simulink model "TosAeb"  (R2021a)
settings: Solver=FixedStepDiscrete  StartTime=0.0  StopTime=inf  FixedStep=0.01  RelTol=1e-3
undefined symbols (not defined in the file or in its folder): ACCModSts, AccSts1, AEB_ObjectMovingOrientation, AEB_ObjectMovingState, CN_BTCActive, CN_CurrentGearR, CN_CurrentGearR_DHT, CN_PTCActive, CN_VDCActive, CN_VSC_EspOff, CN_VSC_FCW_AEB_Error, CN_VSC_FCW_AEB_NoDispay, CN_VSC_FCW_AEB_PerfmDgdtn, CN_VSC_FCW_AEB_TempUnAvlbl, CN_VSC_VehStandstill, CNaccelAvoidMax, CNaccelAvoidMin, CNbrakePedalPress, CNcomfortStrLeftAccelMax, CNcomfortStrLeftAccelMin, CNcomfortStrRightAccelMax, CNcomfortStrRightAccelMin, CNDrvDoorOpen, CNDrvModeEco, CNDrvModeNorm, CNDrvModeOther, CNDrvModeSport, CNDrvSBRreminder, CNNull, CNobjInvalidID, CNPassDoorOpen, CNPassSBRreminder, CNPEBSAccelNegInf, CNPEBSAccelNull, CNPEBSTimeInf, CNPEBSTimeNull, CNSTOPMAX, CNSTOPNULL, CNsubjWidth, CNSwtOFF, CNSwtON, CNTTCMAX, CNTTCNULL, CNVehConfigAEBCycNone, CNVehConfigAEBNone, CNVehConfigAEBPedNone, CNVehConfigFCWNone, CNVehConfigJANone, CollisionSeverity, FALSE, FCWSnvty, JACycObjCalcInfoDefaultParams, JAPedObjCalcInfoDefaultParams, JAVehObjCalcInfoDefaultParams, latCycObjCalcInfoDefaultParams, latPedObjCalcInfoDefaultParams, longObjCalcInfoDefaultParams, ModSts, movingStatus, ObjectType ... (+1112)
libraries: simulink x3031, AEBSFunctionLib x50, Ta_Library x33, AEBSStateMachineLib x23, Mylibrary x12, simulink_extras x7, ObjSelect_lib x3, dspstat3 x2
(part 80 of 102)

== system TosAeb/FcwAebJa_Step_sys/ALG_AEBSystem/ALG_AEBController/AEB_LongTgtInfoCalc/LongObjCalcInfoCalc/AEBS_LongCollisionAssesApp/collisionSeverityHighCalc/collisionSeverityHighCalc1/CollisionAssessHighCalcVersion3/CollisionAssessHighCalc1/CalcBrakePhase3/BrkSysRuntime ==
  a0Obj : Inport
  accelMaxDec : Inport  Port=2
  v0Obj : Inport  Port=3
  v0Ego : Inport  Port=4
  Bus Creator : BusCreator  Inputs=4
  Bus Selector : BusSelector  OutputSignals=a0Obj,accelMaxDec
  Bus Selector1 : BusSelector  OutputSignals=v0Ego,accelMaxDec
  Bus Selector2 : BusSelector  OutputSignals=v0Ego,accelMaxDec,v0Obj,a0Obj
  If : If  IfExpression=u1
  Merge : Merge
  Subsystem : Subsystem
  a0ObjGreatSituation : Subsystem  TreatAsAtomicUnit=on
  a0ObjLessSituation : Subsystem  TreatAsAtomicUnit=on
  tTime : Outport
  wires:
  [a0ObjGreat] -> a0ObjGreatSituation/Action
  If/2 -> [a0ObjGreat]
  Bus Selector2/4 -> a0ObjGreatSituation/4(a0Obj)  "<a0Obj>"
  Bus Selector2/3 -> a0ObjGreatSituation/3(v0Obj)  "<v0Obj>"
  Bus Selector2/2 -> a0ObjGreatSituation/2(accelMaxDec)  "<accelMaxDec>"
  Bus Selector2/1 -> a0ObjGreatSituation/1(vtEgo)  "<v0Ego>"
  Bus Selector1/2 -> a0ObjLessSituation/2(accelMaxDec)  "<accelMaxDec>"
  Bus Selector1/1 -> a0ObjLessSituation/1(vtEgo)  "<v0Ego>"
  Bus Creator/1 -> Bus Selector/1, Bus Selector1/1, Bus Selector2/1
  Bus Selector/2 -> Subsystem/2(accelMaxDec)  "<accelMaxDec>"
  Bus Selector/1 -> Subsystem/1(a0Obj)  "<a0Obj>"
  v0Obj/1 -> Bus Creator/4  "v0Obj"
  v0Ego/1 -> Bus Creator/3  "v0Ego"
  accelMaxDec/1 -> Bus Creator/2  "accelMaxDec"
  Subsystem/1(Flg) -> If/1
  a0Obj/1 -> Bus Creator/1  "a0Obj"
  If/1 -> a0ObjLessSituation/Action
  a0ObjLessSituation/1(tTime) -> Merge/1
  a0ObjGreatSituation/1(tTime) -> Merge/2
  Merge/1 -> tTime/1

== system TosAeb/FcwAebJa_Step_sys/ALG_AEBSystem/ALG_AEBController/AEB_LongTgtInfoCalc/LongObjCalcInfoCalc/AEBS_LongCollisionAssesApp/collisionSeverityHighCalc/collisionSeverityHighCalc1/CollisionAssessHighCalcVersion3/CollisionAssessHighCalc1/CalcBrakePhase3/egoCollisionFlgCalc ==
  stEgo : Inport
  stObj : Inport  Port=2
  dx0 : Inport  Port=3
  Add : Sum
  Compare To Constant : simulink/Logic and Bit Operations/Compare To Constant  relop=<  const=0  OutDataTypeStr=boolean
  Subtract : Sum  Inputs=+-
  egoCollisionFlg : Outport
  wires:
  stObj/1 -> Add/1
  dx0/1 -> Add/2
  Add/1 -> Subtract/1
  stEgo/1 -> Subtract/2
  Subtract/1 -> Compare To Constant/1
  Compare To Constant/1 -> egoCollisionFlg/1

== system TosAeb/FcwAebJa_Step_sys/ALG_AEBSystem/ALG_AEBController/AEB_LongTgtInfoCalc/LongObjCalcInfoCalc/AEBS_LongCollisionAssesApp/collisionSeverityHighCalc/collisionSeverityHighCalc1/CollisionAssessHighCalcVersion3/CollisionAssessHighCalc1/CalcBrakePhase3/stEgoCalc ==
  tTime : Inport
  v0 : Inport  Port=2
  a0 : Inport  Port=3
  Add1 : Sum  Inputs=2
  Constant1 : Constant  Value=0.5
  Product1 : Product  Inputs=4
  Product4 : Product
  st : Outport
  wires:
  tTime/1 -> Product1/4, Product4/1, Product1/3
  Constant1/1 -> Product1/1
  a0/1 -> Product1/2
  v0/1 -> Product4/2
  Product1/1 -> Add1/1
  Product4/1 -> Add1/2
  Add1/1 -> st/1

== system TosAeb/FcwAebJa_Step_sys/ALG_AEBSystem/ALG_AEBController/AEB_LongTgtInfoCalc/LongObjCalcInfoCalc/AEBS_LongCollisionAssesApp/collisionSeverityHighCalc/collisionSeverityHighCalc1/CollisionAssessHighCalcVersion3/CollisionAssessHighCalc1/CalcBrakePhase3/stObjCalc ==
  tTime : Inport
  a0 : Inport  Port=2
  v0 : Inport  Port=3
  Add1 : Sum  Inputs=2
  Constant1 : Constant  Value=0.5
  Product1 : Product  Inputs=4
  Product4 : Product
  st : Outport
  wires:
  Add1/1 -> st/1
  Product4/1 -> Add1/2
  Product1/1 -> Add1/1
  v0/1 -> Product4/2
  a0/1 -> Product1/2
  Constant1/1 -> Product1/1
  tTime/1 -> Product4/1, Product1/4, Product1/3

== system TosAeb/FcwAebJa_Step_sys/ALG_AEBSystem/ALG_AEBController/AEB_LongTgtInfoCalc/LongObjCalcInfoCalc/AEBS_LongCollisionAssesApp/collisionSeverityHighCalc/collisionSeverityHighCalc1/CollisionAssessHighCalcVersion3/CollisionAssessHighCalc1/CalcBrakePhase3/tEgoStopCalc ==
  a0Ego : Inport
  v0Ego : Inport  Port=2
  tStopCalc : Subsystem  TreatAsAtomicUnit=on
  tEgoStop : Outport
  wires:
  v0Ego/1 -> tStopCalc/2(velAbs)
  a0Ego/1 -> tStopCalc/1(accelAbs)
  tStopCalc/1(tStop) -> tEgoStop/1

== system TosAeb/FcwAebJa_Step_sys/ALG_AEBSystem/ALG_AEBController/AEB_LongTgtInfoCalc/LongObjCalcInfoCalc/AEBS_LongCollisionAssesApp/collisionSeverityHighCalc/collisionSeverityHighCalc1/CollisionAssessHighCalcVersion3/CollisionAssessHighCalc1/CalcBrakePhase3/tObjRunCalc ==
  tEgoRun : Inport
  tObjStop : Inport  Port=2
  MinMax : MinMax  Inputs=2
  tObjRun : Outport
  wires:
  MinMax/1 -> tObjRun/1
  tEgoRun/1 -> MinMax/1
  tObjStop/1 -> MinMax/2

== system TosAeb/FcwAebJa_Step_sys/ALG_AEBSystem/ALG_AEBController/AEB_LongTgtInfoCalc/LongObjCalcInfoCalc/AEBS_LongCollisionAssesApp/collisionSeverityHighCalc/collisionSeverityHighCalc1/CollisionAssessHighCalcVersion3/CollisionAssessHighCalc1/CalcBrakePhase3/tObjStopCalc ==
  a0Obj : Inport
  v0Obj : Inport  Port=2
  tStopCalc : Subsystem  TreatAsAtomicUnit=on
  tObjStop : Outport
  wires:
  v0Obj/1 -> tStopCalc/2(velAbs)
  a0Obj/1 -> tStopCalc/1(accelAbs)
  tStopCalc/1(tStop) -> tObjStop/1

== system TosAeb/FcwAebJa_Step_sys/ALG_AEBSystem/ALG_AEBController/AEB_LongTgtInfoCalc/LongObjCalcInfoCalc/AEBS_LongCollisionAssesApp/collisionSeverityHighCalc/collisionSeverityHighCalc1/CollisionAssessHighCalcVersion3/CollisionAssessHighCalc1/CalcBrakePhase3/time4EgoPredic ==
  tEgoStop : Inport
  tEgovDelata : Inport  Port=2
  tTime : Inport  Port=3
  MinMax : MinMax  Inputs=3
  tEgoRun : Outport
  wires:
  tTime/1 -> MinMax/3
  tEgoStop/1 -> MinMax/1
  MinMax/1 -> tEgoRun/1
  tEgovDelata/1 -> MinMax/2

== system TosAeb/FcwAebJa_Step_sys/ALG_AEBSystem/ALG_AEBController/AEB_LongTgtInfoCalc/LongObjCalcInfoCalc/AEBS_LongCollisionAssesApp/collisionSeverityHighCalc/collisionSeverityHighCalc1/CollisionAssessHighCalcVersion3/CollisionAssessHighCalc1/CalcBrakePhase3/timeEgovDeltaCalc ==
  v0Ego : Inport
  velMaxReudce : Inport  Port=2
  a0Ego : Inport  Port=3
  timeEgovDelata : Subsystem  TreatAsAtomicUnit=on
  velMaxReudceFiltCalc : Subsystem
  tEgovDelata : Outport
  wires:
  timeEgovDelata/1(tStop) -> tEgovDelata/1
  a0Ego/1 -> timeEgovDelata/1(accelAbs)
  velMaxReudceFiltCalc/1(velMaxReudceFilt) -> timeEgovDelata/2(velAbs)
  velMaxReudce/1 -> velMaxReudceFiltCalc/2(velMaxReudce)
  v0Ego/1 -> velMaxReudceFiltCalc/1(v0Ego)

== system TosAeb/FcwAebJa_Step_sys/ALG_AEBSystem/ALG_AEBController/AEB_LongTgtInfoCalc/LongObjCalcInfoCalc/AEBS_LongCollisionAssesApp/collisionSeverityHighCalc/collisionSeverityHighCalc1/CollisionAssessHighCalcVersion3/CollisionAssessHighCalc2/CalcBrakePhase1/egoCollisionFlgCalc ==
  stEgo : Inport
  stObj : Inport  Port=2
  dx : Inport  Port=3
  Add : Sum
  Compare To Constant : simulink/Logic and Bit Operations/Compare To Constant  relop=<  const=0  OutDataTypeStr=boolean
  Subtract : Sum  Inputs=+-
  egoCollisionFlg : Outport
  wires:
  stObj/1 -> Add/1
  dx/1 -> Add/2
  Add/1 -> Subtract/1
  stEgo/1 -> Subtract/2
  Subtract/1 -> Compare To Constant/1
  Compare To Constant/1 -> egoCollisionFlg/1

== system TosAeb/FcwAebJa_Step_sys/ALG_AEBSystem/ALG_AEBController/AEB_LongTgtInfoCalc/LongObjCalcInfoCalc/AEBS_LongCollisionAssesApp/collisionSeverityHighCalc/collisionSeverityHighCalc1/CollisionAssessHighCalcVersion3/CollisionAssessHighCalc2/CalcBrakePhase1/restDeltaVelCalc ==
  velMaxReudce : Inport
  v0 : Inport  Port=2
  vt : Inport  Port=3
  Subtract : Sum  Inputs=+-
  Subtract1 : Sum  Inputs=+-
  restDeltaVel : Outport
  wires:
  v0/1 -> Subtract/1
  vt/1 -> Subtract/2
  Subtract/1 -> Subtract1/2
  velMaxReudce/1 -> Subtract1/1
  Subtract1/1 -> restDeltaVel/1

== system TosAeb/FcwAebJa_Step_sys/ALG_AEBSystem/ALG_AEBController/AEB_LongTgtInfoCalc/LongObjCalcInfoCalc/AEBS_LongCollisionAssesApp/collisionSeverityHighCalc/collisionSeverityHighCalc1/CollisionAssessHighCalcVersion3/CollisionAssessHighCalc2/CalcBrakePhase1/stEgoCalc ==
  tTime : Inport
  s0 : Inport  Port=2
  v0 : Inport  Port=3
  a0 : Inport  Port=4
  Add1 : Sum  Inputs=3
  Constant1 : Constant  Value=0.5
  Product1 : Product  Inputs=4
  Product4 : Product
  st : Outport
  wires:
  tTime/1 -> Product1/4, Product4/1, Product1/3
  Constant1/1 -> Product1/1
  a0/1 -> Product1/2
  v0/1 -> Product4/2
  Product1/1 -> Add1/1
  Product4/1 -> Add1/2
  s0/1 -> Add1/3
  Add1/1 -> st/1

== system TosAeb/FcwAebJa_Step_sys/ALG_AEBSystem/ALG_AEBController/AEB_LongTgtInfoCalc/LongObjCalcInfoCalc/AEBS_LongCollisionAssesApp/collisionSeverityHighCalc/collisionSeverityHighCalc1/CollisionAssessHighCalcVersion3/CollisionAssessHighCalc2/CalcBrakePhase1/stObjCalc ==
  tTime : Inport
  a0 : Inport  Port=2
  v0 : Inport  Port=3
  s0 : Inport  Port=4
  Add1 : Sum  Inputs=3
  Constant1 : Constant  Value=0.5
  Product1 : Product  Inputs=4
  Product4 : Product
  st : Outport
  wires:
  Add1/1 -> st/1
  s0/1 -> Add1/3
  Product4/1 -> Add1/2
  Product1/1 -> Add1/1
  v0/1 -> Product4/2
  a0/1 -> Product1/2
  Constant1/1 -> Product1/1
  tTime/1 -> Product4/1, Product1/4, Product1/3

== system TosAeb/FcwAebJa_Step_sys/ALG_AEBSystem/ALG_AEBController/AEB_LongTgtInfoCalc/LongObjCalcInfoCalc/AEBS_LongCollisionAssesApp/collisionSeverityHighCalc/collisionSeverityHighCalc1/CollisionAssessHighCalcVersion3/CollisionAssessHighCalc2/CalcBrakePhase1/tEgoStopCalc ==
  a0Ego : Inport
  v0Ego : Inport  Port=2
  tStopCalc : Subsystem  TreatAsAtomicUnit=on
  tEgoStop : Outport
  wires:
  v0Ego/1 -> tStopCalc/2(velAbs)
  a0Ego/1 -> tStopCalc/1(accelAbs)
  tStopCalc/1(tStop) -> tEgoStop/1

== system TosAeb/FcwAebJa_Step_sys/ALG_AEBSystem/ALG_AEBController/AEB_LongTgtInfoCalc/LongObjCalcInfoCalc/AEBS_LongCollisionAssesApp/collisionSeverityHighCalc/collisionSeverityHighCalc1/CollisionAssessHighCalcVersion3/CollisionAssessHighCalc2/CalcBrakePhase1/tObjRunCalc ==
  tEgoRun : Inport
  tObjStop : Inport  Port=2
  MinMax : MinMax  Inputs=2
  tObjRun : Outport
  wires:
  MinMax/1 -> tObjRun/1
  tEgoRun/1 -> MinMax/1
  tObjStop/1 -> MinMax/2

== system TosAeb/FcwAebJa_Step_sys/ALG_AEBSystem/ALG_AEBController/AEB_LongTgtInfoCalc/LongObjCalcInfoCalc/AEBS_LongCollisionAssesApp/collisionSeverityHighCalc/collisionSeverityHighCalc1/CollisionAssessHighCalcVersion3/CollisionAssessHighCalc2/CalcBrakePhase1/tObjStopCalc ==
  a0Obj : Inport
  v0Obj : Inport  Port=2
  tStopCalc : Subsystem  TreatAsAtomicUnit=on
  tObjStop : Outport
  wires:
  v0Obj/1 -> tStopCalc/2(velAbs)
  a0Obj/1 -> tStopCalc/1(accelAbs)
  tStopCalc/1(tStop) -> tObjStop/1

== system TosAeb/FcwAebJa_Step_sys/ALG_AEBSystem/ALG_AEBController/AEB_LongTgtInfoCalc/LongObjCalcInfoCalc/AEBS_LongCollisionAssesApp/collisionSeverityHighCalc/collisionSeverityHighCalc1/CollisionAssessHighCalcVersion3/CollisionAssessHighCalc2/CalcBrakePhase1/time4EgoPredic ==
  tEgoStop : Inport
  tEgovDelata : Inport  Port=2
  tBrakeLatency : Inport  Port=3
  MinMax : MinMax  Inputs=3
  tEgoRun : Outport
  wires:
  tBrakeLatency/1 -> MinMax/3
  tEgoStop/1 -> MinMax/1
  MinMax/1 -> tEgoRun/1
  tEgovDelata/1 -> MinMax/2

== system TosAeb/FcwAebJa_Step_sys/ALG_AEBSystem/ALG_AEBController/AEB_LongTgtInfoCalc/LongObjCalcInfoCalc/AEBS_LongCollisionAssesApp/collisionSeverityHighCalc/collisionSeverityHighCalc1/CollisionAssessHighCalcVersion3/CollisionAssessHighCalc2/CalcBrakePhase1/timeEgovDelataCalc ==
  v0Ego : Inport
  velMaxReudce : Inport  Port=2
  a0Ego : Inport  Port=3
  timeEgovDelata : Subsystem  TreatAsAtomicUnit=on
  velMaxReudceFiltCalc : Subsystem
  tEgovDelata : Outport
  wires:
  timeEgovDelata/1(tStop) -> tEgovDelata/1
  a0Ego/1 -> timeEgovDelata/1(accelAbs)
  velMaxReudceFiltCalc/1(velMaxReudceFilt) -> timeEgovDelata/2(velAbs)
  velMaxReudce/1 -> velMaxReudceFiltCalc/2(velMaxReudce)
  v0Ego/1 -> velMaxReudceFiltCalc/1(v0Ego)

== system TosAeb/FcwAebJa_Step_sys/ALG_AEBSystem/ALG_AEBController/AEB_LongTgtInfoCalc/LongObjCalcInfoCalc/AEBS_LongCollisionAssesApp/collisionSeverityHighCalc/collisionSeverityHighCalc1/CollisionAssessHighCalcVersion3/CollisionAssessHighCalc2/CalcBrakePhase1/vtEgoCalc ==
  v0 : Inport
  a0 : Inport  Port=2
  tTime : Inport  Port=3
  Add1 : Sum
  Constant1 : Constant  Value=0
  MinMax : MinMax  Function=max  Inputs=2
  Product3 : Product
  vt : Outport
  wires:
  v0/1 -> Add1/2
  Product3/1 -> Add1/1
  a0/1 -> Product3/2
  tTime/1 -> Product3/1
  Add1/1 -> MinMax/1
  MinMax/1 -> vt/1
  Constant1/1 -> MinMax/2

== system TosAeb/FcwAebJa_Step_sys/ALG_AEBSystem/ALG_AEBController/AEB_LongTgtInfoCalc/LongObjCalcInfoCalc/AEBS_LongCollisionAssesApp/collisionSeverityHighCalc/collisionSeverityHighCalc1/CollisionAssessHighCalcVersion3/CollisionAssessHighCalc2/CalcBrakePhase1/vtObjCalc ==
  v0 : Inport
  a0 : Inport  Port=2
  tTime : Inport  Port=3
  Add1 : Sum
  Constant1 : Constant  Value=0
  MinMax : MinMax  Function=max  Inputs=2
  Product3 : Product
  vt : Outport
  wires:
  Constant1/1 -> MinMax/2
  MinMax/1 -> vt/1
  Add1/1 -> MinMax/1
  tTime/1 -> Product3/1
  a0/1 -> Product3/2
  Product3/1 -> Add1/1
  v0/1 -> Add1/2

== system TosAeb/FcwAebJa_Step_sys/ALG_AEBSystem/ALG_AEBController/AEB_LongTgtInfoCalc/LongObjCalcInfoCalc/AEBS_LongCollisionAssesApp/collisionSeverityHighCalc/collisionSeverityHighCalc1/CollisionAssessHighCalcVersion3/CollisionAssessHighCalc2/CalcBrakePhase2/BrkSysRuntimeFhase2 ==
  accelMaxDec : Inport
  a0Ego : Inport  Port=2
  accelGradient : Inport  Port=3
  If : If  IfExpression=u1
  If Action Subsystem : Subsystem  TreatAsAtomicUnit=on
  If Action Subsystem1 : Subsystem  TreatAsAtomicUnit=on
  Merge : Merge
  accelEgoLessAccelmaxReduce : Subsystem
  tTime : Outport
  wires:
  [InvalidCalc] -> If Action Subsystem1/Action
  If/2 -> [InvalidCalc]
  accelEgoLessAccelmaxReduce/1(Flg) -> If/1
  accelMaxDec/1 -> If Action Subsystem/2(accelMaxDec), accelEgoLessAccelmaxReduce/1(accelMaxDec)
  a0Ego/1 -> If Action Subsystem/1(a0Ego), accelEgoLessAccelmaxReduce/2(a0Ego)
  If/1 -> If Action Subsystem/Action
  accelGradient/1 -> If Action Subsystem/3(accelGradient)
  If Action Subsystem/1(tTime) -> Merge/1
  If Action Subsystem1/1(tTime) -> Merge/2
  Merge/1 -> tTime/1

== system TosAeb/FcwAebJa_Step_sys/ALG_AEBSystem/ALG_AEBController/AEB_LongTgtInfoCalc/LongObjCalcInfoCalc/AEBS_LongCollisionAssesApp/collisionSeverityHighCalc/collisionSeverityHighCalc1/CollisionAssessHighCalcVersion3/CollisionAssessHighCalc2/CalcBrakePhase2/Subsystem1 ==
  v0 : Inport
  a0 : Inport  Port=2
  accelGradient : Inport  Port=3
  tTime : Inport  Port=4
  Add : Sum
  Add1 : Sum
  Constant : Constant  Value=0.5
  Constant1 : Constant  Value=0
  MinMax : MinMax  Function=max  Inputs=2
  Product{,1..3} (4 alike) : Product
  vt : Outport
  wires:
  Constant1/1 -> MinMax/2
  MinMax/1 -> vt/1
  Add1/1 -> MinMax/1
  Constant/1 -> Product/1
  accelGradient/1 -> Product/2
  Product/1 -> Product1/1
  tTime/1 -> Product3/1, Product2/2, Product2/1
  Product2/1 -> Product1/2
  a0/1 -> Product3/2
  Product1/1 -> Add/1
  Product3/1 -> Add/2
  Add/1 -> Add1/1
  v0/1 -> Add1/2

== system TosAeb/FcwAebJa_Step_sys/ALG_AEBSystem/ALG_AEBController/AEB_LongTgtInfoCalc/LongObjCalcInfoCalc/AEBS_LongCollisionAssesApp/collisionSeverityHighCalc/collisionSeverityHighCalc1/CollisionAssessHighCalcVersion3/CollisionAssessHighCalc2/CalcBrakePhase2/egoCollisionFlgCalc ==
  stEgo : Inport
  stObj : Inport  Port=2
  dx : Inport  Port=3
  Add : Sum
  Compare To Constant : simulink/Logic and Bit Operations/Compare To Constant  relop=<  const=0  OutDataTypeStr=boolean
  Subtract : Sum  Inputs=+-
  egoCollisionFlg : Outport
  wires:
  stObj/1 -> Add/1
  dx/1 -> Add/2
  Add/1 -> Subtract/1
  stEgo/1 -> Subtract/2
  Subtract/1 -> Compare To Constant/1
  Compare To Constant/1 -> egoCollisionFlg/1

== system TosAeb/FcwAebJa_Step_sys/ALG_AEBSystem/ALG_AEBController/AEB_LongTgtInfoCalc/LongObjCalcInfoCalc/AEBS_LongCollisionAssesApp/collisionSeverityHighCalc/collisionSeverityHighCalc1/CollisionAssessHighCalcVersion3/CollisionAssessHighCalc2/CalcBrakePhase2/restDeltaVelCalc ==
  velMaxReudce : Inport
  v0Ego : Inport  Port=2
  vtEgo : Inport  Port=3
  Subtract : Sum  Inputs=+-
  Subtract1 : Sum  Inputs=+-
  restDeltaVelocity : Outport
  wires:
  Subtract1/1 -> restDeltaVelocity/1
  velMaxReudce/1 -> Subtract1/1
  Subtract/1 -> Subtract1/2
  vtEgo/1 -> Subtract/2
  v0Ego/1 -> Subtract/1

== system TosAeb/FcwAebJa_Step_sys/ALG_AEBSystem/ALG_AEBController/AEB_LongTgtInfoCalc/LongObjCalcInfoCalc/AEBS_LongCollisionAssesApp/collisionSeverityHighCalc/collisionSeverityHighCalc1/CollisionAssessHighCalcVersion3/CollisionAssessHighCalc2/CalcBrakePhase2/stEgoCalc ==
  tTime : Inport
  s0 : Inport  Port=2
  v0 : Inport  Port=3
  a0 : Inport  Port=4
  accelGradient : Inport  Port=5
  Add1 : Sum  Inputs=4
  Constant : Constant  Value=1/6
  Constant1 : Constant  Value=0.5
  Product : Product  Inputs=5
  Product1 : Product  Inputs=4
  Product4 : Product
  st : Outport
  wires:
  Add1/1 -> st/1
  s0/1 -> Add1/4
  Product4/1 -> Add1/3
  Product1/1 -> Add1/2
  Product/1 -> Add1/1
  v0/1 -> Product4/2
  a0/1 -> Product1/3
  Constant1/1 -> Product1/4
  Constant/1 -> Product/1
  accelGradient/1 -> Product/2
  tTime/1 -> Product/3, Product/5, Product1/2, Product4/1, Product1/1, Product/4

== system TosAeb/FcwAebJa_Step_sys/ALG_AEBSystem/ALG_AEBController/AEB_LongTgtInfoCalc/LongObjCalcInfoCalc/AEBS_LongCollisionAssesApp/collisionSeverityHighCalc/collisionSeverityHighCalc1/CollisionAssessHighCalcVersion3/CollisionAssessHighCalc2/CalcBrakePhase2/stObjCalc ==
  tTime : Inport
  a0 : Inport  Port=2
  v0 : Inport  Port=3
  s0 : Inport  Port=4
  Add1 : Sum  Inputs=3
  Constant1 : Constant  Value=0.5
  Product1 : Product  Inputs=4
  Product4 : Product
  st : Outport
  wires:
  Add1/1 -> st/1
  s0/1 -> Add1/3
  Product4/1 -> Add1/2
  Product1/1 -> Add1/1
  v0/1 -> Product4/2
  a0/1 -> Product1/2
  Constant1/1 -> Product1/1
  tTime/1 -> Product4/1, Product1/4, Product1/3

== system TosAeb/FcwAebJa_Step_sys/ALG_AEBSystem/ALG_AEBController/AEB_LongTgtInfoCalc/LongObjCalcInfoCalc/AEBS_LongCollisionAssesApp/collisionSeverityHighCalc/collisionSeverityHighCalc1/CollisionAssessHighCalcVersion3/CollisionAssessHighCalc2/CalcBrakePhase2/tEgoStopCalc ==
  a0Ego : Inport
  v0Ego : Inport  Port=2
  tStopCalc : Subsystem  TreatAsAtomicUnit=on
  tEgoStop : Outport
  wires:
  v0Ego/1 -> tStopCalc/2(velAbs)
  a0Ego/1 -> tStopCalc/1(accelAbs)
  tStopCalc/1(tStop) -> tEgoStop/1

== system TosAeb/FcwAebJa_Step_sys/ALG_AEBSystem/ALG_AEBController/AEB_LongTgtInfoCalc/LongObjCalcInfoCalc/AEBS_LongCollisionAssesApp/collisionSeverityHighCalc/collisionSeverityHighCalc1/CollisionAssessHighCalcVersion3/CollisionAssessHighCalc2/CalcBrakePhase2/tObjRunCalc ==
  tEgoRun : Inport
  tObjStop : Inport  Port=2
  MinMax : MinMax  Inputs=2
  tObjRun : Outport
  wires:
  MinMax/1 -> tObjRun/1
  tEgoRun/1 -> MinMax/1
  tObjStop/1 -> MinMax/2

== system TosAeb/FcwAebJa_Step_sys/ALG_AEBSystem/ALG_AEBController/AEB_LongTgtInfoCalc/LongObjCalcInfoCalc/AEBS_LongCollisionAssesApp/collisionSeverityHighCalc/collisionSeverityHighCalc1/CollisionAssessHighCalcVersion3/CollisionAssessHighCalc2/CalcBrakePhase2/tObjStopCalc ==
  a0Obj : Inport
  v0Obj : Inport  Port=2
  tStopCalc : Subsystem  TreatAsAtomicUnit=on
  tObjStop : Outport
  wires:
  v0Obj/1 -> tStopCalc/2(velAbs)
  a0Obj/1 -> tStopCalc/1(accelAbs)
  tStopCalc/1(tStop) -> tObjStop/1

== system TosAeb/FcwAebJa_Step_sys/ALG_AEBSystem/ALG_AEBController/AEB_LongTgtInfoCalc/LongObjCalcInfoCalc/AEBS_LongCollisionAssesApp/collisionSeverityHighCalc/collisionSeverityHighCalc1/CollisionAssessHighCalcVersion3/CollisionAssessHighCalc2/CalcBrakePhase2/time4EgoPredic ==
  tEgoStop : Inport
  tEgovDelata : Inport  Port=2
  tTime : Inport  Port=3
  MinMax : MinMax  Inputs=3
  tEgoRun : Outport
  wires:
  tTime/1 -> MinMax/3
  tEgoStop/1 -> MinMax/1
  MinMax/1 -> tEgoRun/1
  tEgovDelata/1 -> MinMax/2

== system TosAeb/FcwAebJa_Step_sys/ALG_AEBSystem/ALG_AEBController/AEB_LongTgtInfoCalc/LongObjCalcInfoCalc/AEBS_LongCollisionAssesApp/collisionSeverityHighCalc/collisionSeverityHighCalc1/CollisionAssessHighCalcVersion3/CollisionAssessHighCalc2/CalcBrakePhase2/timeEgovDelataCalc ==
  v0Ego : Inport
  velMaxReudce : Inport  Port=2
  a0Ego : Inport  Port=3
  timeEgovDelata : Subsystem  TreatAsAtomicUnit=on
  velMaxReudceFiltCalc : Subsystem
  tEgovDelata : Outport
  wires:
  timeEgovDelata/1(tStop) -> tEgovDelata/1
  a0Ego/1 -> timeEgovDelata/1(accelAbs)
  velMaxReudceFiltCalc/1(velMaxReudceFilt) -> timeEgovDelata/2(velAbs)
  velMaxReudce/1 -> velMaxReudceFiltCalc/2(velMaxReudce)
  v0Ego/1 -> velMaxReudceFiltCalc/1(v0Ego)

== system TosAeb/FcwAebJa_Step_sys/ALG_AEBSystem/ALG_AEBController/AEB_LongTgtInfoCalc/LongObjCalcInfoCalc/AEBS_LongCollisionAssesApp/collisionSeverityHighCalc/collisionSeverityHighCalc1/CollisionAssessHighCalcVersion3/CollisionAssessHighCalc2/CalcBrakePhase2/vtObjCalc ==
  v0 : Inport
  a0 : Inport  Port=2
  tTime : Inport  Port=3
  Add1 : Sum
  Constant1 : Constant  Value=0
  MinMax : MinMax  Function=max  Inputs=2
  Product3 : Product
  vt : Outport
  wires:
  Constant1/1 -> MinMax/2
  MinMax/1 -> vt/1
  Add1/1 -> MinMax/1
  tTime/1 -> Product3/1
  a0/1 -> Product3/2
  Product3/1 -> Add1/1
  v0/1 -> Add1/2

== system TosAeb/FcwAebJa_Step_sys/ALG_AEBSystem/ALG_AEBController/AEB_LongTgtInfoCalc/LongObjCalcInfoCalc/AEBS_LongCollisionAssesApp/collisionSeverityHighCalc/collisionSeverityHighCalc1/CollisionAssessHighCalcVersion3/CollisionAssessHighCalc2/CalcBrakePhase3/BrkSysRuntime ==
  a0Obj : Inport
  accelMaxDec : Inport  Port=2
  v0Obj : Inport  Port=3
  v0Ego : Inport  Port=4
  Bus Creator : BusCreator  Inputs=4
  Bus Selector : BusSelector  OutputSignals=a0Obj,accelMaxDec
  Bus Selector1 : BusSelector  OutputSignals=v0Ego,accelMaxDec
  Bus Selector2 : BusSelector  OutputSignals=v0Ego,accelMaxDec,v0Obj,a0Obj
  If : If  IfExpression=u1
  Merge : Merge
  Subsystem : Subsystem
  a0ObjGreatSituation : Subsystem  TreatAsAtomicUnit=on
  a0ObjLessSituation : Subsystem  TreatAsAtomicUnit=on
  tTime : Outport
  wires:
  [a0ObjGreat] -> a0ObjGreatSituation/Action
  If/2 -> [a0ObjGreat]
  Bus Selector2/4 -> a0ObjGreatSituation/4(a0Obj)  "<a0Obj>"
  Bus Selector2/3 -> a0ObjGreatSituation/3(v0Obj)  "<v0Obj>"
  Bus Selector2/2 -> a0ObjGreatSituation/2(accelMaxDec)  "<accelMaxDec>"
  Bus Selector2/1 -> a0ObjGreatSituation/1(vtEgo)  "<v0Ego>"
  Bus Selector1/2 -> a0ObjLessSituation/2(accelMaxDec)  "<accelMaxDec>"
  Bus Selector1/1 -> a0ObjLessSituation/1(vtEgo)  "<v0Ego>"
  Bus Creator/1 -> Bus Selector/1, Bus Selector1/1, Bus Selector2/1
  Bus Selector/2 -> Subsystem/2(accelMaxDec)  "<accelMaxDec>"
  Bus Selector/1 -> Subsystem/1(a0Obj)  "<a0Obj>"
  v0Obj/1 -> Bus Creator/4  "v0Obj"
  v0Ego/1 -> Bus Creator/3  "v0Ego"
  accelMaxDec/1 -> Bus Creator/2  "accelMaxDec"
  Subsystem/1(Flg) -> If/1
  a0Obj/1 -> Bus Creator/1  "a0Obj"
  If/1 -> a0ObjLessSituation/Action
  a0ObjLessSituation/1(tTime) -> Merge/1
  a0ObjGreatSituation/1(tTime) -> Merge/2
  Merge/1 -> tTime/1

== system TosAeb/FcwAebJa_Step_sys/ALG_AEBSystem/ALG_AEBController/AEB_LongTgtInfoCalc/LongObjCalcInfoCalc/AEBS_LongCollisionAssesApp/collisionSeverityHighCalc/collisionSeverityHighCalc1/CollisionAssessHighCalcVersion3/CollisionAssessHighCalc2/CalcBrakePhase3/egoCollisionFlgCalc ==
  stEgo : Inport
  stObj : Inport  Port=2
  dx : Inport  Port=3
  Add : Sum
  Compare To Constant : simulink/Logic and Bit Operations/Compare To Constant  relop=<  const=0  OutDataTypeStr=boolean
  Subtract : Sum  Inputs=+-
  egoCollisionFlg : Outport
  wires:
  stObj/1 -> Add/1
  dx/1 -> Add/2
  Add/1 -> Subtract/1
  stEgo/1 -> Subtract/2
  Subtract/1 -> Compare To Constant/1
  Compare To Constant/1 -> egoCollisionFlg/1

== system TosAeb/FcwAebJa_Step_sys/ALG_AEBSystem/ALG_AEBController/AEB_LongTgtInfoCalc/LongObjCalcInfoCalc/AEBS_LongCollisionAssesApp/collisionSeverityHighCalc/collisionSeverityHighCalc1/CollisionAssessHighCalcVersion3/CollisionAssessHighCalc2/CalcBrakePhase3/stEgoCalc ==
  tTime : Inport
  s0 : Inport  Port=2
  v0 : Inport  Port=3
  a0 : Inport  Port=4
  Add1 : Sum  Inputs=3
  Constant1 : Constant  Value=0.5
  Product1 : Product  Inputs=4
  Product4 : Product
  st : Outport
  wires:
  tTime/1 -> Product1/4, Product4/1, Product1/3
  Constant1/1 -> Product1/1
  a0/1 -> Product1/2
  v0/1 -> Product4/2
  Product1/1 -> Add1/1
  Product4/1 -> Add1/2
  s0/1 -> Add1/3
  Add1/1 -> st/1

== system TosAeb/FcwAebJa_Step_sys/ALG_AEBSystem/ALG_AEBController/AEB_LongTgtInfoCalc/LongObjCalcInfoCalc/AEBS_LongCollisionAssesApp/collisionSeverityHighCalc/collisionSeverityHighCalc1/CollisionAssessHighCalcVersion3/CollisionAssessHighCalc2/CalcBrakePhase3/stObjCalc ==
  tTime : Inport
  a0 : Inport  Port=2
  v0 : Inport  Port=3
  s0 : Inport  Port=4
  Add1 : Sum  Inputs=3
  Constant1 : Constant  Value=0.5
  Product1 : Product  Inputs=4
  Product4 : Product
  st : Outport
  wires:
  Add1/1 -> st/1
  s0/1 -> Add1/3
  Product4/1 -> Add1/2
  Product1/1 -> Add1/1
  v0/1 -> Product4/2
  a0/1 -> Product1/2
  Constant1/1 -> Product1/1
  tTime/1 -> Product4/1, Product1/4, Product1/3

== system TosAeb/FcwAebJa_Step_sys/ALG_AEBSystem/ALG_AEBController/AEB_LongTgtInfoCalc/LongObjCalcInfoCalc/AEBS_LongCollisionAssesApp/collisionSeverityHighCalc/collisionSeverityHighCalc1/CollisionAssessHighCalcVersion3/CollisionAssessHighCalc2/CalcBrakePhase3/tEgoStopCalc ==
  a0Ego : Inport
  v0Ego : Inport  Port=2
  tStopCalc : Subsystem  TreatAsAtomicUnit=on
  tEgoStop : Outport
  wires:
  v0Ego/1 -> tStopCalc/2(velAbs)
  a0Ego/1 -> tStopCalc/1(accelAbs)
  tStopCalc/1(tStop) -> tEgoStop/1

== system TosAeb/FcwAebJa_Step_sys/ALG_AEBSystem/ALG_AEBController/AEB_LongTgtInfoCalc/LongObjCalcInfoCalc/AEBS_LongCollisionAssesApp/collisionSeverityHighCalc/collisionSeverityHighCalc1/CollisionAssessHighCalcVersion3/CollisionAssessHighCalc2/CalcBrakePhase3/tObjRunCalc ==
  tEgoRun : Inport
  tObjStop : Inport  Port=2
  MinMax : MinMax  Inputs=2
  tObjRun : Outport
  wires:
  MinMax/1 -> tObjRun/1
  tEgoRun/1 -> MinMax/1
  tObjStop/1 -> MinMax/2

== system TosAeb/FcwAebJa_Step_sys/ALG_AEBSystem/ALG_AEBController/AEB_LongTgtInfoCalc/LongObjCalcInfoCalc/AEBS_LongCollisionAssesApp/collisionSeverityHighCalc/collisionSeverityHighCalc1/CollisionAssessHighCalcVersion3/CollisionAssessHighCalc2/CalcBrakePhase3/tObjStopCalc ==
  a0Obj : Inport
  v0Obj : Inport  Port=2
  tStopCalc : Subsystem  TreatAsAtomicUnit=on
  tObjStop : Outport
  wires:
  v0Obj/1 -> tStopCalc/2(velAbs)
  a0Obj/1 -> tStopCalc/1(accelAbs)
  tStopCalc/1(tStop) -> tObjStop/1

== system TosAeb/FcwAebJa_Step_sys/ALG_AEBSystem/ALG_AEBController/AEB_LongTgtInfoCalc/LongObjCalcInfoCalc/AEBS_LongCollisionAssesApp/collisionSeverityHighCalc/collisionSeverityHighCalc1/CollisionAssessHighCalcVersion3/CollisionAssessHighCalc2/CalcBrakePhase3/time4EgoPredic ==
  tEgoStop : Inport
  tEgovDelata : Inport  Port=2
  tTime : Inport  Port=3
  MinMax : MinMax  Inputs=3
  tEgoRun : Outport
  wires:
  tTime/1 -> MinMax/3
  tEgoStop/1 -> MinMax/1
  MinMax/1 -> tEgoRun/1
  tEgovDelata/1 -> MinMax/2

== system TosAeb/FcwAebJa_Step_sys/ALG_AEBSystem/ALG_AEBController/AEB_LongTgtInfoCalc/LongObjCalcInfoCalc/AEBS_LongCollisionAssesApp/collisionSeverityHighCalc/collisionSeverityHighCalc1/CollisionAssessHighCalcVersion3/CollisionAssessHighCalc2/CalcBrakePhase3/timeEgovDelataCalc ==
  v0Ego : Inport
  velMaxReudce : Inport  Port=2
  a0Ego : Inport  Port=3
  timeEgovDelata : Subsystem  TreatAsAtomicUnit=on
  velMaxReudceFiltCalc : Subsystem
  tEgovDelata : Outport
  wires:
  timeEgovDelata/1(tStop) -> tEgovDelata/1
  a0Ego/1 -> timeEgovDelata/1(accelAbs)
  velMaxReudceFiltCalc/1(velMaxReudceFilt) -> timeEgovDelata/2(velAbs)
  velMaxReudce/1 -> velMaxReudceFiltCalc/2(velMaxReudce)
  v0Ego/1 -> velMaxReudceFiltCalc/1(v0Ego)

== system TosAeb/FcwAebJa_Step_sys/ALG_AEBSystem/ALG_AEBController/AEB_LongTgtInfoCalc/LongObjCalcInfoCalc/AEBS_LongCollisionAssesApp/collisionSeverityHighCalc/collisionSeverityHighCalc2/collisionSeverityHighPhase1/Phase1TimeCalc/tEgoStopWorkPhase1/TimeEgoStopCalc ==
  subjLongAccel : Inport
  subjVel : Inport  Port=2
  TimeEgoStopCalcPort : ActionPort
  Divide : Product  Inputs=*/
  Gain : Gain  Gain=-1
  tEgoStop : Outport
  wires:
  Divide/1 -> tEgoStop/1  "tEgoStop"
  subjVel/1 -> Divide/1  "subjVel"
  subjLongAccel/1 -> Gain/1  "subjAccel"
  Gain/1 -> Divide/2

== system TosAeb/FcwAebJa_Step_sys/ALG_AEBSystem/ALG_AEBController/AEB_LongTgtInfoCalc/LongObjCalcInfoCalc/AEBS_LongCollisionAssesApp/collisionSeverityHighCalc/collisionSeverityHighCalc2/collisionSeverityHighPhase1/Phase1TimeCalc/tEgoStopWorkPhase1/TimeEgoStopInf ==
  Action Port : ActionPort
  Constant : Constant  Value=CNPEBSTimeInf
  tEgoStop : Outport
  wires:
  Constant/1 -> tEgoStop/1

== system TosAeb/FcwAebJa_Step_sys/ALG_AEBSystem/ALG_AEBController/AEB_LongTgtInfoCalc/LongObjCalcInfoCalc/AEBS_LongCollisionAssesApp/collisionSeverityHighCalc/collisionSeverityHighCalc2/collisionSeverityHighPhase1/Phase1TimeCalc/tMaxReducePhase1Calc/TimeMaxReduceCalc ==
  note: velMaxBrakeReduced
      | tMaxReduce = - -----------------------------------
      | 					subjAccel
  note: if velMaxReudce < subjVel
      | 	velMaxBrakeReduced = velMaxReudce;
      | else
      | 	velMaxBrakeReduced = subjAccel;
  subjLongAccel : Inport
  velMaxReudce : Inport  Port=2
  subjVel : Inport  Port=3
  Action Port : ActionPort
  Divide : Product  Inputs=*/
  Gain : Gain  Gain=-1
  Relational Operator : RelationalOperator  Operator=<  OutDataTypeStr=boolean  RndMeth=Simplest
  Switch : Switch  Criteria=u2 ~= 0
  tMaxReduce : Outport
  wires:
  Divide/1 -> tMaxReduce/1  "tMaxReduce"
  subjLongAccel/1 -> Gain/1  "subjAccel"
  Gain/1 -> Divide/2
  Switch/1 -> Divide/1
  velMaxReudce/1 -> Relational Operator/1, Switch/1  "velMaxReudce"
  subjVel/1 -> Switch/3, Relational Operator/2  "subjVel"
  Relational Operator/1 -> Switch/2

== system TosAeb/FcwAebJa_Step_sys/ALG_AEBSystem/ALG_AEBController/AEB_LongTgtInfoCalc/LongObjCalcInfoCalc/AEBS_LongCollisionAssesApp/collisionSeverityHighCalc/collisionSeverityHighCalc2/collisionSeverityHighPhase1/Phase1TimeCalc/tMaxReducePhase1Calc/TimeMaxReduceInf ==
  Action Port : ActionPort
  Constant : Constant  Value=CNPEBSTimeInf
  tMaxReduce : Outport
  wires:
  Constant/1 -> tMaxReduce/1

== system TosAeb/FcwAebJa_Step_sys/ALG_AEBSystem/ALG_AEBController/AEB_LongTgtInfoCalc/LongObjCalcInfoCalc/AEBS_LongCollisionAssesApp/collisionSeverityHighCalc/collisionSeverityHighCalc2/collisionSeverityHighPhase1/Phase1TimeCalc/tObjStopCalc/StopTimeFlow/tStopFuncCalc ==
  velRel : Inport
  accelRel : Inport  Port=2
  tStopFuncCalc : TriggerPort  TriggerType=function-call  VariantControl=Variant
      settings: CopyFcn=Stateflow.SLINSF.SimulinkMan.removeBlockPreDeleteFcn  PreDeleteFcn=Stateflow.SLINSF.SimulinkMan.unsafePredeleteFcn
  Abs : Abs
  Abs1 : Abs
  Divide : Product  Inputs=*/
  temptStop : Outport
  wires:
  accelRel/1 -> Abs1/1
  Divide/1 -> temptStop/1
  Abs1/1 -> Divide/2
  Abs/1 -> Divide/1
  velRel/1 -> Abs/1

== system TosAeb/FcwAebJa_Step_sys/ALG_AEBSystem/ALG_AEBController/AEB_LongTgtInfoCalc/LongObjCalcInfoCalc/AEBS_LongCollisionAssesApp/collisionSeverityHighCalc/collisionSeverityHighCalc2/collisionSeverityHighPhase2/Phase2TimeCalc/TimeEgoStopCalc/TimeEgoStopCalc ==
  note: subjVelWorkPhase1
      | tEgoStop = - ----------------------------
      | 			subjAccelWorkPhase1
  subjVelWorkPhase1 : Inport
  subjLongAccelWorkPhase1 : Inport  Port=2
  Action Port : ActionPort
  Divide : Product  Inputs=*/
  Gain : Gain  Gain=-1
  tEgoStop : Outport
  wires:
  Divide/1 -> tEgoStop/1
  subjVelWorkPhase1/1 -> Divide/1
  subjLongAccelWorkPhase1/1 -> Gain/1
  Gain/1 -> Divide/2

== system TosAeb/FcwAebJa_Step_sys/ALG_AEBSystem/ALG_AEBController/AEB_LongTgtInfoCalc/LongObjCalcInfoCalc/AEBS_LongCollisionAssesApp/collisionSeverityHighCalc/collisionSeverityHighCalc2/collisionSeverityHighPhase2/Phase2TimeCalc/TimeEgoStopCalc/TimeEgoStopInf ==
  Action Port : ActionPort
  Constant : Constant  Value=CNPEBSTimeInf
  tEgoStop : Outport
  wires:
  Constant/1 -> tEgoStop/1  "CNPEBSTimeInf"

== system TosAeb/FcwAebJa_Step_sys/ALG_AEBSystem/ALG_AEBController/AEB_LongTgtInfoCalc/LongObjCalcInfoCalc/AEBS_LongCollisionAssesApp/collisionSeverityHighCalc/collisionSeverityHighCalc2/collisionSeverityHighPhase2/Phase2TimeCalc/TimeGradientPhase2Calc/If Action Subsystem6 ==
  Action Port : ActionPort
  Constant : Constant  Value=CNPEBSTimeNull
  tGradientPhase2 : Outport
  wires:
  Constant/1 -> tGradientPhase2/1  "CNPEBSTimeNull"

== system TosAeb/FcwAebJa_Step_sys/ALG_AEBSystem/ALG_AEBController/AEB_LongTgtInfoCalc/LongObjCalcInfoCalc/AEBS_LongCollisionAssesApp/collisionSeverityHighCalc/collisionSeverityHighCalc2/collisionSeverityHighPhase2/Phase2TimeCalc/TimeGradientPhase2Calc/If Action Subsystem7 ==
  note: (accelMaxReudce - subjAccelWorkPhase1)
      | tGradientPhase2 = ------------------------------------------------------------------
      | 							accelGradient
  accelMaxReudce : Inport
  subjLongAccelWorkPhase1 : Inport  Port=2
  accelGradient : Inport  Port=3
  Action Port : ActionPort
  Divide : Product  Inputs=*/
  Subtract : Sum  Inputs=+-
  tGradientPhase2 : Outport
  wires:
  Subtract/1 -> Divide/1
  accelGradient/1 -> Divide/2
  Divide/1 -> tGradientPhase2/1
  accelMaxReudce/1 -> Subtract/1
  subjLongAccelWorkPhase1/1 -> Subtract/2

== system TosAeb/FcwAebJa_Step_sys/ALG_AEBSystem/ALG_AEBController/AEB_LongTgtInfoCalc/LongObjCalcInfoCalc/AEBS_LongCollisionAssesApp/collisionSeverityHighCalc/collisionSeverityHighCalc2/collisionSeverityHighPhase2/Phase2TimeCalc/tMaxReducePhase2Calc/TimeMaxReduceCalc ==
  note: velMaxBrakeReudce
      | tMaxReduce = - -------------------------------
      | 				subjAccelWorkPhase1
  note: if  velMaxReudce < subjVelWorkPhase1
      | 	 velMaxBrakeReudce = velMaxReudce;
      | else
      | 	velMaxBrakeReudce = subjVelWorkPhase1;
  subjLongAccelWorkPhase1 : Inport
  velMaxReudce : Inport  Port=2
  subjVelWorkPhase1 : Inport  Port=3
  Action Port : ActionPort
  Divide : Product  Inputs=*/
  Gain : Gain  Gain=-1
  Relational Operator : RelationalOperator  Operator=<  OutDataTypeStr=boolean  RndMeth=Simplest
  Switch : Switch  Criteria=u2 ~= 0
  tMaxReduce : Outport
  wires:
  Divide/1 -> tMaxReduce/1
  subjLongAccelWorkPhase1/1 -> Gain/1
  Gain/1 -> Divide/2
  Switch/1 -> Divide/1
  velMaxReudce/1 -> Relational Operator/1, Switch/1
  subjVelWorkPhase1/1 -> Switch/3, Relational Operator/2
  Relational Operator/1 -> Switch/2

== system TosAeb/FcwAebJa_Step_sys/ALG_AEBSystem/ALG_AEBController/AEB_LongTgtInfoCalc/LongObjCalcInfoCalc/AEBS_LongCollisionAssesApp/collisionSeverityHighCalc/collisionSeverityHighCalc2/collisionSeverityHighPhase2/Phase2TimeCalc/tMaxReducePhase2Calc/TimeMaxReduceInf ==
  Action Port : ActionPort
  Constant : Constant  Value=CNPEBSTimeInf
  tMaxReduce : Outport
  wires:
  Constant/1 -> tMaxReduce/1  "CNPEBSTimeInf"

== system TosAeb/FcwAebJa_Step_sys/ALG_AEBSystem/ALG_AEBController/AEB_LongTgtInfoCalc/LongObjCalcInfoCalc/AEBS_LongCollisionAssesApp/collisionSeverityHighCalc/collisionSeverityHighCalc2/collisionSeverityHighPhase2/Phase2TimeCalc/tObjStopCalc/StopTimeFlow/tStopFuncCalc ==
  velRel : Inport
  accelRel : Inport  Port=2
  tStopFuncCalc : TriggerPort  TriggerType=function-call  VariantControl=Variant
      settings: CopyFcn=Stateflow.SLINSF.SimulinkMan.removeBlockPreDeleteFcn  PreDeleteFcn=Stateflow.SLINSF.SimulinkMan.unsafePredeleteFcn
  Abs : Abs
  Abs1 : Abs
  Divide : Product  Inputs=*/
  temptStop : Outport
  wires:
  accelRel/1 -> Abs1/1
  Divide/1 -> temptStop/1
  Abs1/1 -> Divide/2
  Abs/1 -> Divide/1
  velRel/1 -> Abs/1

== system TosAeb/FcwAebJa_Step_sys/ALG_AEBSystem/ALG_AEBController/AEB_LongTgtInfoCalc/LongObjCalcInfoCalc/AEBS_LongCollisionAssesApp/collisionSeverityHighCalc/collisionSeverityHighCalc2/collisionSeverityHighPhase3/Phase3TimeCalc/TimeEgoStopCalc/TimeEgoStopCalc ==
  note: subjVelWorkPhase2
      | tEgoStop = - ----------------------------
      | 			subjAccelWorkPhase2
  accelMaxReduce : Inport
  subjVelWorkPhase2 : Inport  Port=2
  Action Port : ActionPort
  Divide : Product  Inputs=*/
  Gain : Gain  Gain=-1
  tEgoStop : Outport
  wires:
  Divide/1 -> tEgoStop/1
  subjVelWorkPhase2/1 -> Divide/1
  accelMaxReduce/1 -> Gain/1
  Gain/1 -> Divide/2

== system TosAeb/FcwAebJa_Step_sys/ALG_AEBSystem/ALG_AEBController/AEB_LongTgtInfoCalc/LongObjCalcInfoCalc/AEBS_LongCollisionAssesApp/collisionSeverityHighCalc/collisionSeverityHighCalc2/collisionSeverityHighPhase3/Phase3TimeCalc/TimeEgoStopCalc/TimeEgoStopInf ==
  Action Port : ActionPort
  Constant : Constant  Value=CNPEBSTimeInf
  tEgoStop : Outport
  wires:
  Constant/1 -> tEgoStop/1

== system TosAeb/FcwAebJa_Step_sys/ALG_AEBSystem/ALG_AEBController/AEB_LongTgtInfoCalc/LongObjCalcInfoCalc/AEBS_LongCollisionAssesApp/collisionSeverityHighCalc/collisionSeverityHighCalc2/collisionSeverityHighPhase3/Phase3TimeCalc/tBrakeConstCalc/tBrakeConstCalcSituation1 ==
  note: subjVelWorkPhase2
      | tBrakeConst = ---------------------------------
      | 				-accelMaxReduce
  subjVelWorkPhase2 : Inport
  accelMaxReduce : Inport  Port=2
  function : TriggerPort  TriggerType=function-call  VariantControl=Variant
  Divide : Product  Inputs=*/
  Gain : Gain  Gain=-1
  tBrakeConst : Outport
  wires:
  Gain/1 -> Divide/2
  subjVelWorkPhase2/1 -> Divide/1
  accelMaxReduce/1 -> Gain/1
  Divide/1 -> tBrakeConst/1

== system TosAeb/FcwAebJa_Step_sys/ALG_AEBSystem/ALG_AEBController/AEB_LongTgtInfoCalc/LongObjCalcInfoCalc/AEBS_LongCollisionAssesApp/collisionSeverityHighCalc/collisionSeverityHighCalc2/collisionSeverityHighPhase3/Phase3TimeCalc/tBrakeConstCalc/tBrakeConstCalcSituation2 ==
  note: subjVelWorkPhase2 - vxAbsWorkPhase2Target
      | tBrakeConst = - --------------------------------------------------------------
      | 				accelMaxReduce - axAbsWorkPhase2Target
  subjVelWorkPhase2 : Inport
  vxAbsWorkPhase2Target : Inport  Port=2
  accelMaxReduce : Inport  Port=3
  axAbsWorkPhase2Target : Inport  Port=4
  function : TriggerPort  TriggerType=function-call  VariantControl=Variant
  Divide : Product  Inputs=*/
  Gain : Gain  Gain=-1
  Subtract : Sum  Inputs=+-
  Subtract1 : Sum  Inputs=+-
  tBrakeConst : Outport
  wires:
  Gain/1 -> Divide/2
  Divide/1 -> tBrakeConst/1
  accelMaxReduce/1 -> Subtract/1
  axAbsWorkPhase2Target/1 -> Subtract/2
  subjVelWorkPhase2/1 -> Subtract1/1
  vxAbsWorkPhase2Target/1 -> Subtract1/2
  Subtract1/1 -> Divide/1
  Subtract/1 -> Gain/1

== system TosAeb/FcwAebJa_Step_sys/ALG_AEBSystem/ALG_AEBController/AEB_LongTgtInfoCalc/LongObjCalcInfoCalc/AEBS_LongCollisionAssesApp/collisionSeverityHighCalc/collisionSeverityHighCalc2/collisionSeverityHighPhase3/Phase3TimeCalc/tMaxReduceCalc/TimeMaxReduceCalc ==
  note: velMaxBrakeReduce
      | tMaxReduce = - -------------------------------
      | 					subjAccel
  note: if velMaxReduce < subjVel
      | 	velMaxBrakeReduce = velMaxReduce;
      | else
      | 	velMaxBrakeReduce = subjVel;
  subjLongAccelWorkPhase2 : Inport
  velMaxReudce : Inport  Port=2
  subjVelWorkPhase2 : Inport  Port=3
  Action Port : ActionPort
  Divide : Product  Inputs=*/
  Gain : Gain  Gain=-1
  Relational Operator : RelationalOperator  Operator=<  OutDataTypeStr=boolean  RndMeth=Simplest
  Switch : Switch  Criteria=u2 ~= 0
  tMaxReduce : Outport
  wires:
  Divide/1 -> tMaxReduce/1
  subjLongAccelWorkPhase2/1 -> Gain/1
  Gain/1 -> Divide/2
  Switch/1 -> Divide/1
  velMaxReudce/1 -> Relational Operator/1, Switch/1
  subjVelWorkPhase2/1 -> Switch/3, Relational Operator/2
  Relational Operator/1 -> Switch/2

== system TosAeb/FcwAebJa_Step_sys/ALG_AEBSystem/ALG_AEBController/AEB_LongTgtInfoCalc/LongObjCalcInfoCalc/AEBS_LongCollisionAssesApp/collisionSeverityHighCalc/collisionSeverityHighCalc2/collisionSeverityHighPhase3/Phase3TimeCalc/tMaxReduceCalc/TimeMaxReduceInf ==
  Action Port : ActionPort
  Constant : Constant  Value=CNPEBSTimeInf
  tMaxReduce : Outport
  wires:
  Constant/1 -> tMaxReduce/1  "CNPEBSTimeInf"

== system TosAeb/FcwAebJa_Step_sys/ALG_AEBSystem/ALG_AEBController/AEB_LongTgtInfoCalc/LongObjCalcInfoCalc/AEBS_LongCollisionAssesApp/collisionSeverityHighCalc/collisionSeverityHighCalc2/collisionSeverityHighPhase3/Phase3TimeCalc/tObjStopCalc/StopTimeFlow/tStopFuncCalc ==
  velRel : Inport
  accelRel : Inport  Port=2
  tStopFuncCalc : TriggerPort  TriggerType=function-call  VariantControl=Variant
      settings: CopyFcn=Stateflow.SLINSF.SimulinkMan.removeBlockPreDeleteFcn  PreDeleteFcn=Stateflow.SLINSF.SimulinkMan.unsafePredeleteFcn
  Abs : Abs
  Abs1 : Abs
  Divide : Product  Inputs=*/
  temptStop : Outport
  wires:
  accelRel/1 -> Abs1/1
  Divide/1 -> temptStop/1
  Abs1/1 -> Divide/2
  Abs/1 -> Divide/1
  velRel/1 -> Abs/1

== system TosAeb/AEB_Objsel/TA_Processor/AEB_ObjSelect/TargetInfoCheck/Data_precess/TA_EffectCalculate/Obj_FilterFlgCalculate/JA_ObjFilter/CollisionProbCalc/Subsystem/PreCrossFlgCalcFlow/VectorCrossAlgorithm/If Action Subsystem1/Subsystem ==
  temp_u : Inport  OutDataTypeStr=single
  temp_t : Inport  Port=2  OutDataTypeStr=single
  vectorMulit : Inport  Port=3
  Compare To Constant : simulink/Logic and Bit Operations/Compare To Constant  relop=>=  const=0  OutDataTypeStr=boolean
  Compare To Constant1 : simulink/Logic and Bit Operations/Compare To Constant  relop=<=  const=1  OutDataTypeStr=boolean
  Compare To Constant2 : simulink/Logic and Bit Operations/Compare To Constant  relop=>=  const=0  OutDataTypeStr=boolean
  Compare To Constant3 : simulink/Logic and Bit Operations/Compare To Constant  relop=<=  const=1  OutDataTypeStr=boolean
  Divide : Product  Inputs=*/
  Divide1 : Product  Inputs=*/
  Logical Operator : Logic  Inputs=4  AllPortsSameDT=off  OutDataTypeStr=boolean
  CrossFlg : Outport
  wires:
  vectorMulit/1 -> Divide1/2, Divide/2
  temp_t/1 -> Divide/1
  temp_u/1 -> Divide1/1
  Divide1/1 -> Compare To Constant1/1, Compare To Constant/1
  Divide/1 -> Compare To Constant3/1, Compare To Constant2/1
  Compare To Constant1/1 -> Logical Operator/2
  Compare To Constant2/1 -> Logical Operator/3
  Compare To Constant/1 -> Logical Operator/1
  Compare To Constant3/1 -> Logical Operator/4
  Logical Operator/1 -> CrossFlg/1

== system TosAeb/AEB_Objsel/TA_Processor/AEB_ObjSelect/TargetInfoCheck/Data_precess/TA_EffectCalculate/Obj_FilterFlgCalculate/JA_ObjFilter/CollisionProbCalc/Subsystem/PreCrossFlgCalcFlow/VectorCrossAlgorithm/If Action Subsystem1/t ==
  m2 : Inport  OutDataTypeStr=single
  m1 : Inport  Port=2  OutDataTypeStr=single
  y1 : Inport  Port=3  OutDataTypeStr=single
  n1 : Inport  Port=4  OutDataTypeStr=single
  n2 : Inport  Port=5  OutDataTypeStr=single
  x1 : Inport  Port=6  OutDataTypeStr=single
  Product : Product  OutDataTypeStr=single
  Product1 : Product  OutDataTypeStr=single
  Subtract{1..5} (5 alike) : Sum  Inputs=+-  OutDataTypeStr=single
  temp_t : Outport
  wires:
  m2/1 -> Subtract2/1
  m1/1 -> Subtract2/2, Subtract1/2
  y1/1 -> Subtract3/1
  Subtract2/1 -> Product/1
  Subtract3/1 -> Product/2
  n2/1 -> Subtract4/1
  n1/1 -> Subtract4/2, Subtract3/2
  x1/1 -> Subtract1/1
  Subtract4/1 -> Product1/1
  Subtract1/1 -> Product1/2
  Product/1 -> Subtract5/1
  Product1/1 -> Subtract5/2
  Subtract5/1 -> temp_t/1

== system TosAeb/AEB_Objsel/TA_Processor/AEB_ObjSelect/TargetInfoCheck/Data_precess/TA_EffectCalculate/Obj_FilterFlgCalculate/JA_ObjFilter/CollisionProbCalc/Subsystem/PreCrossFlgCalcFlow/VectorCrossAlgorithm/If Action Subsystem1/u ==
  x2 : Inport  OutDataTypeStr=single
  x1 : Inport  Port=2  OutDataTypeStr=single
  y1 : Inport  Port=3  OutDataTypeStr=single
  n1 : Inport  Port=4  OutDataTypeStr=single
  y2 : Inport  Port=5  OutDataTypeStr=single
  m1 : Inport  Port=6  OutDataTypeStr=single
  Product : Product  OutDataTypeStr=single
  Product1 : Product  OutDataTypeStr=single
  Subtract{1..5} (5 alike) : Sum  Inputs=+-  OutDataTypeStr=single
  temp_u : Outport
  wires:
  Product1/1 -> Subtract5/2
  Product/1 -> Subtract5/1
  Subtract1/1 -> Product1/2
  Subtract4/1 -> Product1/1
  n1/1 -> Subtract3/2
  y2/1 -> Subtract4/1
  Subtract3/1 -> Product/2
  Subtract2/1 -> Product/1
  y1/1 -> Subtract4/2, Subtract3/1
  x1/1 -> Subtract1/1, Subtract2/2
  x2/1 -> Subtract2/1
  m1/1 -> Subtract1/2
  Subtract5/1 -> temp_u/1

== system TosAeb/AEB_Objsel/TA_Processor/AEB_ObjSelect/TargetInfoCheck/Data_precess/TA_EffectCalculate/Obj_FilterFlgCalculate/Lat_ObjFilter/Lat_Objelse/Lat_ObjPre/Subsystem/LatDis_Cal/L2R ==
  dy : Inport
  Width_Large : Inport  Port=2
  Width_half : Inport  Port=3
  dx : Inport  Port=4
  ax : Inport  Port=5
  ay : Inport  Port=6
  vx : Inport  Port=7
  vy : Inport  Port=8
  Action Port : ActionPort  ActionPortLabel=if(u1 > 0)
  Constant : Constant  Value=P_TA_PrmForVehLongth
  L2R_Calculate : MATLAB Function  TreatAsAtomicUnit=on
  L2R_Calculate1 : MATLAB Function  Commented=on  TreatAsAtomicUnit=on
  Valid_Flg : Outport
  TTI : Outport  Port=2
  wires:
  vx/1 -> L2R_Calculate/6(vx)
  ay/1 -> L2R_Calculate/5(ay)
  vy/1 -> L2R_Calculate/7(vy)
  dy/1 -> L2R_Calculate/1(dy)
  ax/1 -> L2R_Calculate/4(ax)
  Width_Large/1 -> L2R_Calculate/2(Width_Large)
  dx/1 -> L2R_Calculate/3(dx)
  Width_half/1 -> L2R_Calculate1/3(Width_half)
  L2R_Calculate/1(Valid_Flg) -> Valid_Flg/1
  L2R_Calculate/2(TTI) -> TTI/1
  Constant/1 -> L2R_Calculate1/9(P_TA_PrmForVehLongth)

== system TosAeb/AEB_Objsel/TA_Processor/AEB_ObjSelect/TargetInfoCheck/Data_precess/TA_EffectCalculate/Obj_FilterFlgCalculate/Lat_ObjFilter/Lat_Objelse/Lat_ObjPre/Subsystem/LatDis_Cal/Obj_PosnJudge ==
  Oreintation : Inport
  vy : Inport  Port=2
  Compare To Constant2 : simulink/Logic and Bit Operations/Compare To Constant  relop===  const=3  OutDataTypeStr=boolean
  Compare To Constant3 : simulink/Logic and Bit Operations/Compare To Constant  relop=>  const=P_TA_PrmForLatObjLatSpdThred  OutDataTypeStr=boolean
  Compare To Constant4 : simulink/Logic and Bit Operations/Compare To Constant  relop===  const=2  OutDataTypeStr=boolean
  Compare To Constant5 : simulink/Logic and Bit Operations/Compare To Constant  relop=>  const=P_TA_PrmForLatObjLatSpdThred  OutDataTypeStr=boolean
  Data Type Conversion : DataTypeConversion
  Data Type Conversion1 : DataTypeConversion
  Gain1 : Gain  Gain=-1  OutDataTypeStr=single
  Logical Operator : Logic  AllPortsSameDT=off  OutDataTypeStr=boolean
  Logical Operator1 : Logic  AllPortsSameDT=off  OutDataTypeStr=boolean
  Scope : Scope  NumInputPorts=2
  L2R : Outport
  R2L : Outport  Port=2
  wires:
  Oreintation/1 -> Compare To Constant4/1, Compare To Constant2/1
  Compare To Constant2/1 -> Logical Operator/1
  Compare To Constant5/1 -> Logical Operator1/2
  Compare To Constant4/1 -> Logical Operator1/1
  vy/1 -> Compare To Constant5/1, Gain1/1
  Compare To Constant3/1 -> Logical Operator/2
  Logical Operator1/1 -> Data Type Conversion1/1
  Logical Operator/1 -> Data Type Conversion/1
  Data Type Conversion/1 -> Scope/1, L2R/1  "L2R"
  Data Type Conversion1/1 -> Scope/2, R2L/1  "R2L"
  Gain1/1 -> Compare To Constant3/1

== system TosAeb/AEB_Objsel/TA_Processor/AEB_ObjSelect/TargetInfoCheck/Data_precess/TA_EffectCalculate/Obj_FilterFlgCalculate/Lat_ObjFilter/Lat_Objelse/Lat_ObjPre/Subsystem/LatDis_Cal/R2L ==
  dy : Inport
  Width_Large : Inport  Port=2
  Width_half : Inport  Port=3
  dx : Inport  Port=4
  ax : Inport  Port=5
  ay : Inport  Port=6
  vx : Inport  Port=7
  vy : Inport  Port=8
  Action Port : ActionPort  ActionPortLabel=elseif(u2 > 0)
  Constant : Constant  Value=P_TA_PrmForVehLongth
  R2L_Calculate : MATLAB Function  TreatAsAtomicUnit=on
  R2L_Calculate1 : MATLAB Function  Commented=on  TreatAsAtomicUnit=on
  Signal Conversion : SignalConversion  OverrideOpt=off
  Valid_Flg : Outport
  TTI : Outport  Port=2
  wires:
  Width_half/1 -> R2L_Calculate1/3(Width_half)
  Width_Large/1 -> R2L_Calculate/2(Width_Large)
  ax/1 -> R2L_Calculate/4(ax)  "ax"
  dy/1 -> R2L_Calculate/1(dy)  "dy"
  vy/1 -> R2L_Calculate/7(vy)  "vy"
  ay/1 -> R2L_Calculate/5(ay)  "ay"
  vx/1 -> R2L_Calculate/6(vx)  "vx"
  R2L_Calculate/1(Valid_Flg) -> Valid_Flg/1
  R2L_Calculate/2(TTI) -> Signal Conversion/1
  Constant/1 -> R2L_Calculate1/9(P_TA_PrmForVehLongth)
  Signal Conversion/1 -> TTI/1
  dx/1 -> R2L_Calculate/3(dx)  "dx"
